simulink model "chapter2_5"  (R2022a) (continued)
== system chapter2_5/Subsystem3/Subsystem/Subsystem3/Subsystem13 ==
  index : Inport
  F : Inport  Port=2
  Add1 : Sum
  Add2 : Sum
  Constant2 : Constant
  Constant6 : Constant
  Constant7 : Constant
  Constant8 : Constant  Value=2
  Demux : Demux  Outputs=2
  Mux : Mux  Inputs=2
  Subsystem : Subsystem
  Subsystem1 : Subsystem
  Subsystem2 : Subsystem
  Subsystem3 : Subsystem
  Out1 : Outport
  wires:
  Mux/1 -> Out1/1
  Subsystem2/2(Out2) -> Add2/1
  Subsystem3/2(Out2) -> Add2/2
  Add2/1 -> Mux/2
  Subsystem3/1(Out1) -> Add1/2
  Subsystem2/1(Out1) -> Add1/1
  Subsystem1/8(Omiga_n) -> Subsystem3/8(Omiga_n)
  Subsystem1/7(Omiga_bn) -> Subsystem3/7(Omiga_bn)
  Subsystem1/6(static_n) -> Subsystem3/6(static_n)
  Subsystem1/5(Sn) -> Subsystem3/5(Sn)
  Subsystem1/4(theta_n3) -> Subsystem3/4(theta_n3)
  Subsystem1/3(theta_n2) -> Subsystem3/3(theta_n2)
  Subsystem1/2(theta_n1) -> Subsystem3/2(theta_n1)
  Subsystem1/1(An1) -> Subsystem3/1(An1)
  Subsystem/8(Omiga_n) -> Subsystem2/8(Omiga_n)
  Subsystem/7(Omiga_bn) -> Subsystem2/7(Omiga_bn)
  Subsystem/6(static_n) -> Subsystem2/6(static_n)
  Subsystem/5(Sn) -> Subsystem2/5(Sn)
  Subsystem/1(An1) -> Subsystem2/1(An1)
  Subsystem/4(theta_n3) -> Subsystem2/4(theta_n3)
  Subsystem/3(theta_n2) -> Subsystem2/3(theta_n2)
  Subsystem/2(theta_n1) -> Subsystem2/2(theta_n1)
  Demux/2 -> Subsystem1/4(F)
  Demux/1 -> Subsystem/4(F)
  F/1 -> Demux/1
  Constant7/1 -> Subsystem1/2(r)
  Constant8/1 -> Subsystem1/3(k)
  Constant6/1 -> Subsystem/3(k)
  Constant2/1 -> Subsystem/2(r)
  Add1/1 -> Mux/1
  index/1 -> Subsystem/1(imod), Subsystem1/1(imod)

== system chapter2_5/Subsystem3/Subsystem/Subsystem3/Subsystem14 ==
  index : Inport
  F : Inport  Port=2
  Add1 : Sum
  Add2 : Sum
  Constant2 : Constant
  Constant6 : Constant
  Constant7 : Constant
  Constant8 : Constant  Value=2
  Demux : Demux  Outputs=2
  Mux : Mux  Inputs=2
  Subsystem : Subsystem
  Subsystem1 : Subsystem
  Subsystem2 : Subsystem
  Subsystem3 : Subsystem
  Out1 : Outport
  wires:
  index/1 -> Subsystem1/1(imod), Subsystem/1(imod)
  Add1/1 -> Mux/1
  Constant2/1 -> Subsystem/2(r)
  Constant6/1 -> Subsystem/3(k)
  Constant8/1 -> Subsystem1/3(k)
  Constant7/1 -> Subsystem1/2(r)
  F/1 -> Demux/1
  Demux/1 -> Subsystem/4(F)
  Demux/2 -> Subsystem1/4(F)
  Subsystem/2(theta_n1) -> Subsystem2/2(theta_n1)
  Subsystem/3(theta_n2) -> Subsystem2/3(theta_n2)
  Subsystem/4(theta_n3) -> Subsystem2/4(theta_n3)
  Subsystem/1(An1) -> Subsystem2/1(An1)
  Subsystem/5(Sn) -> Subsystem2/5(Sn)
  Subsystem/6(static_n) -> Subsystem2/6(static_n)
  Subsystem/7(Omiga_bn) -> Subsystem2/7(Omiga_bn)
  Subsystem/8(Omiga_n) -> Subsystem2/8(Omiga_n)
  Subsystem1/1(An1) -> Subsystem3/1(An1)
  Subsystem1/2(theta_n1) -> Subsystem3/2(theta_n1)
  Subsystem1/3(theta_n2) -> Subsystem3/3(theta_n2)
  Subsystem1/4(theta_n3) -> Subsystem3/4(theta_n3)
  Subsystem1/5(Sn) -> Subsystem3/5(Sn)
  Subsystem1/6(static_n) -> Subsystem3/6(static_n)
  Subsystem1/7(Omiga_bn) -> Subsystem3/7(Omiga_bn)
  Subsystem1/8(Omiga_n) -> Subsystem3/8(Omiga_n)
  Subsystem2/1(Out1) -> Add1/1
  Subsystem3/1(Out1) -> Add1/2
  Add2/1 -> Mux/2
  Subsystem3/2(Out2) -> Add2/2
  Subsystem2/2(Out2) -> Add2/1
  Mux/1 -> Out1/1

== system chapter2_5/Subsystem3/Subsystem/Subsystem3/Subsystem15 ==
  index : Inport
  F : Inport  Port=2
  Add1 : Sum
  Add2 : Sum
  Constant2 : Constant
  Constant6 : Constant
  Constant7 : Constant
  Constant8 : Constant  Value=2
  Demux : Demux  Outputs=2
  Mux : Mux  Inputs=2
  Subsystem : Subsystem
  Subsystem1 : Subsystem
  Subsystem2 : Subsystem
  Subsystem3 : Subsystem
  Out1 : Outport
  wires:
  index/1 -> Subsystem1/1(imod), Subsystem/1(imod)
  Add1/1 -> Mux/1
  Constant2/1 -> Subsystem/2(r)
  Constant6/1 -> Subsystem/3(k)
  Constant8/1 -> Subsystem1/3(k)
  Constant7/1 -> Subsystem1/2(r)
  F/1 -> Demux/1
  Demux/1 -> Subsystem/4(F)
  Demux/2 -> Subsystem1/4(F)
  Subsystem/2(theta_n1) -> Subsystem2/2(theta_n1)
  Subsystem/3(theta_n2) -> Subsystem2/3(theta_n2)
  Subsystem/4(theta_n3) -> Subsystem2/4(theta_n3)
  Subsystem/1(An1) -> Subsystem2/1(An1)
  Subsystem/5(Sn) -> Subsystem2/5(Sn)
  Subsystem/6(static_n) -> Subsystem2/6(static_n)
  Subsystem/7(Omiga_bn) -> Subsystem2/7(Omiga_bn)
  Subsystem/8(Omiga_n) -> Subsystem2/8(Omiga_n)
  Subsystem1/1(An1) -> Subsystem3/1(An1)
  Subsystem1/2(theta_n1) -> Subsystem3/2(theta_n1)
  Subsystem1/3(theta_n2) -> Subsystem3/3(theta_n2)
  Subsystem1/4(theta_n3) -> Subsystem3/4(theta_n3)
  Subsystem1/5(Sn) -> Subsystem3/5(Sn)
  Subsystem1/6(static_n) -> Subsystem3/6(static_n)
  Subsystem1/7(Omiga_bn) -> Subsystem3/7(Omiga_bn)
  Subsystem1/8(Omiga_n) -> Subsystem3/8(Omiga_n)
  Subsystem2/1(Out1) -> Add1/1
  Subsystem3/1(Out1) -> Add1/2
  Add2/1 -> Mux/2
  Subsystem3/2(Out2) -> Add2/2
  Subsystem2/2(Out2) -> Add2/1
  Mux/1 -> Out1/1

== system chapter2_5/Subsystem3/Subsystem/Subsystem3/Subsystem16 ==
  index : Inport
  F : Inport  Port=2
  Add1 : Sum
  Add2 : Sum
  Constant2 : Constant
  Constant6 : Constant
  Constant7 : Constant
  Constant8 : Constant  Value=2
  Demux : Demux  Outputs=2
  Mux : Mux  Inputs=2
  Subsystem : Subsystem
  Subsystem1 : Subsystem
  Subsystem2 : Subsystem
  Subsystem3 : Subsystem
  Out1 : Outport
  wires:
  Mux/1 -> Out1/1
  Subsystem2/2(Out2) -> Add2/1
  Subsystem3/2(Out2) -> Add2/2
  Add2/1 -> Mux/2
  Subsystem3/1(Out1) -> Add1/2
  Subsystem2/1(Out1) -> Add1/1
  Subsystem1/8(Omiga_n) -> Subsystem3/8(Omiga_n)
  Subsystem1/7(Omiga_bn) -> Subsystem3/7(Omiga_bn)
  Subsystem1/6(static_n) -> Subsystem3/6(static_n)
  Subsystem1/5(Sn) -> Subsystem3/5(Sn)
  Subsystem1/4(theta_n3) -> Subsystem3/4(theta_n3)
  Subsystem1/3(theta_n2) -> Subsystem3/3(theta_n2)
  Subsystem1/2(theta_n1) -> Subsystem3/2(theta_n1)
  Subsystem1/1(An1) -> Subsystem3/1(An1)
  Subsystem/8(Omiga_n) -> Subsystem2/8(Omiga_n)
  Subsystem/7(Omiga_bn) -> Subsystem2/7(Omiga_bn)
  Subsystem/6(static_n) -> Subsystem2/6(static_n)
  Subsystem/5(Sn) -> Subsystem2/5(Sn)
  Subsystem/1(An1) -> Subsystem2/1(An1)
  Subsystem/4(theta_n3) -> Subsystem2/4(theta_n3)
  Subsystem/3(theta_n2) -> Subsystem2/3(theta_n2)
  Subsystem/2(theta_n1) -> Subsystem2/2(theta_n1)
  Demux/2 -> Subsystem1/4(F)
  Demux/1 -> Subsystem/4(F)
  F/1 -> Demux/1
  Constant7/1 -> Subsystem1/2(r)
  Constant8/1 -> Subsystem1/3(k)
  Constant6/1 -> Subsystem/3(k)
  Constant2/1 -> Subsystem/2(r)
  Add1/1 -> Mux/1
  index/1 -> Subsystem/1(imod), Subsystem1/1(imod)

== system chapter2_5/Subsystem3/Subsystem/Subsystem3/Subsystem17 ==
  index : Inport
  F : Inport  Port=2
  Add1 : Sum
  Add2 : Sum
  Constant2 : Constant
  Constant6 : Constant
  Constant7 : Constant
  Constant8 : Constant  Value=2
  Demux : Demux  Outputs=2
  Mux : Mux  Inputs=2
  Subsystem : Subsystem
  Subsystem1 : Subsystem
  Subsystem2 : Subsystem
  Subsystem3 : Subsystem
  Out1 : Outport
  wires:
  Mux/1 -> Out1/1
  Subsystem2/2(Out2) -> Add2/1
  Subsystem3/2(Out2) -> Add2/2
  Add2/1 -> Mux/2
  Subsystem3/1(Out1) -> Add1/2
  Subsystem2/1(Out1) -> Add1/1
  Subsystem1/8(Omiga_n) -> Subsystem3/8(Omiga_n)
  Subsystem1/7(Omiga_bn) -> Subsystem3/7(Omiga_bn)
  Subsystem1/6(static_n) -> Subsystem3/6(static_n)
  Subsystem1/5(Sn) -> Subsystem3/5(Sn)
  Subsystem1/4(theta_n3) -> Subsystem3/4(theta_n3)
  Subsystem1/3(theta_n2) -> Subsystem3/3(theta_n2)
  Subsystem1/2(theta_n1) -> Subsystem3/2(theta_n1)
  Subsystem1/1(An1) -> Subsystem3/1(An1)
  Subsystem/8(Omiga_n) -> Subsystem2/8(Omiga_n)
  Subsystem/7(Omiga_bn) -> Subsystem2/7(Omiga_bn)
  Subsystem/6(static_n) -> Subsystem2/6(static_n)
  Subsystem/5(Sn) -> Subsystem2/5(Sn)
  Subsystem/1(An1) -> Subsystem2/1(An1)
  Subsystem/4(theta_n3) -> Subsystem2/4(theta_n3)
  Subsystem/3(theta_n2) -> Subsystem2/3(theta_n2)
  Subsystem/2(theta_n1) -> Subsystem2/2(theta_n1)
  Demux/2 -> Subsystem1/4(F)
  Demux/1 -> Subsystem/4(F)
  F/1 -> Demux/1
  Constant7/1 -> Subsystem1/2(r)
  Constant8/1 -> Subsystem1/3(k)
  Constant6/1 -> Subsystem/3(k)
  Constant2/1 -> Subsystem/2(r)
  Add1/1 -> Mux/1
  index/1 -> Subsystem/1(imod), Subsystem1/1(imod)

== system chapter2_5/Subsystem3/Subsystem/Subsystem3/Subsystem18 ==
  index : Inport
  F : Inport  Port=2
  Add1 : Sum
  Add2 : Sum
  Constant2 : Constant
  Constant6 : Constant
  Constant7 : Constant
  Constant8 : Constant  Value=2
  Demux : Demux  Outputs=2
  Mux : Mux  Inputs=2
  Subsystem : Subsystem
  Subsystem1 : Subsystem
  Subsystem2 : Subsystem
  Subsystem3 : Subsystem
  Out1 : Outport
  wires:
  index/1 -> Subsystem1/1(imod), Subsystem/1(imod)
  Add1/1 -> Mux/1
  Constant2/1 -> Subsystem/2(r)
  Constant6/1 -> Subsystem/3(k)
  Constant8/1 -> Subsystem1/3(k)
  Constant7/1 -> Subsystem1/2(r)
  F/1 -> Demux/1
  Demux/1 -> Subsystem/4(F)
  Demux/2 -> Subsystem1/4(F)
  Subsystem/2(theta_n1) -> Subsystem2/2(theta_n1)
  Subsystem/3(theta_n2) -> Subsystem2/3(theta_n2)
  Subsystem/4(theta_n3) -> Subsystem2/4(theta_n3)
  Subsystem/1(An1) -> Subsystem2/1(An1)
  Subsystem/5(Sn) -> Subsystem2/5(Sn)
  Subsystem/6(static_n) -> Subsystem2/6(static_n)
  Subsystem/7(Omiga_bn) -> Subsystem2/7(Omiga_bn)
  Subsystem/8(Omiga_n) -> Subsystem2/8(Omiga_n)
  Subsystem1/1(An1) -> Subsystem3/1(An1)
  Subsystem1/2(theta_n1) -> Subsystem3/2(theta_n1)
  Subsystem1/3(theta_n2) -> Subsystem3/3(theta_n2)
  Subsystem1/4(theta_n3) -> Subsystem3/4(theta_n3)
  Subsystem1/5(Sn) -> Subsystem3/5(Sn)
  Subsystem1/6(static_n) -> Subsystem3/6(static_n)
  Subsystem1/7(Omiga_bn) -> Subsystem3/7(Omiga_bn)
  Subsystem1/8(Omiga_n) -> Subsystem3/8(Omiga_n)
  Subsystem2/1(Out1) -> Add1/1
  Subsystem3/1(Out1) -> Add1/2
  Add2/1 -> Mux/2
  Subsystem3/2(Out2) -> Add2/2
  Subsystem2/2(Out2) -> Add2/1
  Mux/1 -> Out1/1

== system chapter2_5/Subsystem3/Subsystem/Subsystem3/Subsystem19 ==
  index : Inport
  F : Inport  Port=2
  Add1 : Sum
  Add2 : Sum
  Constant2 : Constant
  Constant6 : Constant
  Constant7 : Constant
  Constant8 : Constant  Value=2
  Demux : Demux  Outputs=2
  Mux : Mux  Inputs=2
  Subsystem : Subsystem
  Subsystem1 : Subsystem
  Subsystem2 : Subsystem
  Subsystem3 : Subsystem
  Out1 : Outport
  wires:
  Mux/1 -> Out1/1
  Subsystem2/2(Out2) -> Add2/1
  Subsystem3/2(Out2) -> Add2/2
  Add2/1 -> Mux/2
  Subsystem3/1(Out1) -> Add1/2
  Subsystem2/1(Out1) -> Add1/1
  Subsystem1/8(Omiga_n) -> Subsystem3/8(Omiga_n)
  Subsystem1/7(Omiga_bn) -> Subsystem3/7(Omiga_bn)
  Subsystem1/6(static_n) -> Subsystem3/6(static_n)
  Subsystem1/5(Sn) -> Subsystem3/5(Sn)
  Subsystem1/4(theta_n3) -> Subsystem3/4(theta_n3)
  Subsystem1/3(theta_n2) -> Subsystem3/3(theta_n2)
  Subsystem1/2(theta_n1) -> Subsystem3/2(theta_n1)
  Subsystem1/1(An1) -> Subsystem3/1(An1)
  Subsystem/8(Omiga_n) -> Subsystem2/8(Omiga_n)
  Subsystem/7(Omiga_bn) -> Subsystem2/7(Omiga_bn)
  Subsystem/6(static_n) -> Subsystem2/6(static_n)
  Subsystem/5(Sn) -> Subsystem2/5(Sn)
  Subsystem/1(An1) -> Subsystem2/1(An1)
  Subsystem/4(theta_n3) -> Subsystem2/4(theta_n3)
  Subsystem/3(theta_n2) -> Subsystem2/3(theta_n2)
  Subsystem/2(theta_n1) -> Subsystem2/2(theta_n1)
  Demux/2 -> Subsystem1/4(F)
  Demux/1 -> Subsystem/4(F)
  F/1 -> Demux/1
  Constant7/1 -> Subsystem1/2(r)
  Constant8/1 -> Subsystem1/3(k)
  Constant6/1 -> Subsystem/3(k)
  Constant2/1 -> Subsystem/2(r)
  Add1/1 -> Mux/1
  index/1 -> Subsystem/1(imod), Subsystem1/1(imod)

== system chapter2_5/Subsystem3/Subsystem/Subsystem3/Subsystem2 ==
  index : Inport
  F : Inport  Port=2
  Add1 : Sum
  Add2 : Sum
  Constant2 : Constant
  Constant6 : Constant
  Constant7 : Constant
  Constant8 : Constant  Value=2
  Demux : Demux  Outputs=2
  Mux : Mux  Inputs=2
  Subsystem : Subsystem
  Subsystem1 : Subsystem
  Subsystem2 : Subsystem
  Subsystem3 : Subsystem
  Out1 : Outport
  wires:
  Mux/1 -> Out1/1
  Subsystem2/2(Out2) -> Add2/1
  Subsystem3/2(Out2) -> Add2/2
  Add2/1 -> Mux/2
  Subsystem3/1(Out1) -> Add1/2
  Subsystem2/1(Out1) -> Add1/1
  Subsystem1/8(Omiga_n) -> Subsystem3/8(Omiga_n)
  Subsystem1/7(Omiga_bn) -> Subsystem3/7(Omiga_bn)
  Subsystem1/6(static_n) -> Subsystem3/6(static_n)
  Subsystem1/5(Sn) -> Subsystem3/5(Sn)
  Subsystem1/4(theta_n3) -> Subsystem3/4(theta_n3)
  Subsystem1/3(theta_n2) -> Subsystem3/3(theta_n2)
  Subsystem1/2(theta_n1) -> Subsystem3/2(theta_n1)
  Subsystem1/1(An1) -> Subsystem3/1(An1)
  Subsystem/8(Omiga_n) -> Subsystem2/8(Omiga_n)
  Subsystem/7(Omiga_bn) -> Subsystem2/7(Omiga_bn)
  Subsystem/6(static_n) -> Subsystem2/6(static_n)
  Subsystem/5(Sn) -> Subsystem2/5(Sn)
  Subsystem/1(An1) -> Subsystem2/1(An1)
  Subsystem/4(theta_n3) -> Subsystem2/4(theta_n3)
  Subsystem/3(theta_n2) -> Subsystem2/3(theta_n2)
  Subsystem/2(theta_n1) -> Subsystem2/2(theta_n1)
  Demux/2 -> Subsystem1/4(F)
  Demux/1 -> Subsystem/4(F)
  F/1 -> Demux/1
  Constant7/1 -> Subsystem1/2(r)
  Constant8/1 -> Subsystem1/3(k)
  Constant6/1 -> Subsystem/3(k)
  Constant2/1 -> Subsystem/2(r)
  Add1/1 -> Mux/1
  index/1 -> Subsystem/1(imod), Subsystem1/1(imod)

== system chapter2_5/Subsystem3/Subsystem/Subsystem3/Subsystem20 ==
  index : Inport
  F : Inport  Port=2
  Add1 : Sum
  Add2 : Sum
  Constant2 : Constant
  Constant6 : Constant
  Constant7 : Constant
  Constant8 : Constant  Value=2
  Demux : Demux  Outputs=2
  Mux : Mux  Inputs=2
  Subsystem : Subsystem
  Subsystem1 : Subsystem
  Subsystem2 : Subsystem
  Subsystem3 : Subsystem
  Out1 : Outport
  wires:
  index/1 -> Subsystem1/1(imod), Subsystem/1(imod)
  Add1/1 -> Mux/1
  Constant2/1 -> Subsystem/2(r)
  Constant6/1 -> Subsystem/3(k)
  Constant8/1 -> Subsystem1/3(k)
  Constant7/1 -> Subsystem1/2(r)
  F/1 -> Demux/1
  Demux/1 -> Subsystem/4(F)
  Demux/2 -> Subsystem1/4(F)
  Subsystem/2(theta_n1) -> Subsystem2/2(theta_n1)
  Subsystem/3(theta_n2) -> Subsystem2/3(theta_n2)
  Subsystem/4(theta_n3) -> Subsystem2/4(theta_n3)
  Subsystem/1(An1) -> Subsystem2/1(An1)
  Subsystem/5(Sn) -> Subsystem2/5(Sn)
  Subsystem/6(static_n) -> Subsystem2/6(static_n)
  Subsystem/7(Omiga_bn) -> Subsystem2/7(Omiga_bn)
  Subsystem/8(Omiga_n) -> Subsystem2/8(Omiga_n)
  Subsystem1/1(An1) -> Subsystem3/1(An1)
  Subsystem1/2(theta_n1) -> Subsystem3/2(theta_n1)
  Subsystem1/3(theta_n2) -> Subsystem3/3(theta_n2)
  Subsystem1/4(theta_n3) -> Subsystem3/4(theta_n3)
  Subsystem1/5(Sn) -> Subsystem3/5(Sn)
  Subsystem1/6(static_n) -> Subsystem3/6(static_n)
  Subsystem1/7(Omiga_bn) -> Subsystem3/7(Omiga_bn)
  Subsystem1/8(Omiga_n) -> Subsystem3/8(Omiga_n)
  Subsystem2/1(Out1) -> Add1/1
  Subsystem3/1(Out1) -> Add1/2
  Add2/1 -> Mux/2
  Subsystem3/2(Out2) -> Add2/2
  Subsystem2/2(Out2) -> Add2/1
  Mux/1 -> Out1/1

== system chapter2_5/Subsystem3/Subsystem/Subsystem3/Subsystem3 ==
  index : Inport
  F : Inport  Port=2
  Add1 : Sum
  Add2 : Sum
  Constant2 : Constant
  Constant6 : Constant
  Constant7 : Constant
  Constant8 : Constant  Value=2
  Demux : Demux  Outputs=2
  Mux : Mux  Inputs=2
  Subsystem : Subsystem
  Subsystem1 : Subsystem
  Subsystem2 : Subsystem
  Subsystem3 : Subsystem
  Out1 : Outport
  wires:
  index/1 -> Subsystem1/1(imod), Subsystem/1(imod)
  Add1/1 -> Mux/1
  Constant2/1 -> Subsystem/2(r)
  Constant6/1 -> Subsystem/3(k)
  Constant8/1 -> Subsystem1/3(k)
  Constant7/1 -> Subsystem1/2(r)
  F/1 -> Demux/1
  Demux/1 -> Subsystem/4(F)
  Demux/2 -> Subsystem1/4(F)
  Subsystem/2(theta_n1) -> Subsystem2/2(theta_n1)
  Subsystem/3(theta_n2) -> Subsystem2/3(theta_n2)
  Subsystem/4(theta_n3) -> Subsystem2/4(theta_n3)
  Subsystem/1(An1) -> Subsystem2/1(An1)
  Subsystem/5(Sn) -> Subsystem2/5(Sn)
  Subsystem/6(static_n) -> Subsystem2/6(static_n)
  Subsystem/7(Omiga_bn) -> Subsystem2/7(Omiga_bn)
  Subsystem/8(Omiga_n) -> Subsystem2/8(Omiga_n)
  Subsystem1/1(An1) -> Subsystem3/1(An1)
  Subsystem1/2(theta_n1) -> Subsystem3/2(theta_n1)
  Subsystem1/3(theta_n2) -> Subsystem3/3(theta_n2)
  Subsystem1/4(theta_n3) -> Subsystem3/4(theta_n3)
  Subsystem1/5(Sn) -> Subsystem3/5(Sn)
  Subsystem1/6(static_n) -> Subsystem3/6(static_n)
  Subsystem1/7(Omiga_bn) -> Subsystem3/7(Omiga_bn)
  Subsystem1/8(Omiga_n) -> Subsystem3/8(Omiga_n)
  Subsystem2/1(Out1) -> Add1/1
  Subsystem3/1(Out1) -> Add1/2
  Add2/1 -> Mux/2
  Subsystem3/2(Out2) -> Add2/2
  Subsystem2/2(Out2) -> Add2/1
  Mux/1 -> Out1/1

== system chapter2_5/Subsystem3/Subsystem/Subsystem3/Subsystem4 ==
  index : Inport
  F : Inport  Port=2
  Add1 : Sum
  Add2 : Sum
  Constant2 : Constant
  Constant6 : Constant
  Constant7 : Constant
  Constant8 : Constant  Value=2
  Demux : Demux  Outputs=2
  Mux : Mux  Inputs=2
  Subsystem : Subsystem
  Subsystem1 : Subsystem
  Subsystem2 : Subsystem
  Subsystem3 : Subsystem
  Out1 : Outport
  wires:
  Mux/1 -> Out1/1
  Subsystem2/2(Out2) -> Add2/1
  Subsystem3/2(Out2) -> Add2/2
  Add2/1 -> Mux/2
  Subsystem3/1(Out1) -> Add1/2
  Subsystem2/1(Out1) -> Add1/1
  Subsystem1/8(Omiga_n) -> Subsystem3/8(Omiga_n)
  Subsystem1/7(Omiga_bn) -> Subsystem3/7(Omiga_bn)
  Subsystem1/6(static_n) -> Subsystem3/6(static_n)
  Subsystem1/5(Sn) -> Subsystem3/5(Sn)
  Subsystem1/4(theta_n3) -> Subsystem3/4(theta_n3)
  Subsystem1/3(theta_n2) -> Subsystem3/3(theta_n2)
  Subsystem1/2(theta_n1) -> Subsystem3/2(theta_n1)
  Subsystem1/1(An1) -> Subsystem3/1(An1)
  Subsystem/8(Omiga_n) -> Subsystem2/8(Omiga_n)
  Subsystem/7(Omiga_bn) -> Subsystem2/7(Omiga_bn)
  Subsystem/6(static_n) -> Subsystem2/6(static_n)
  Subsystem/5(Sn) -> Subsystem2/5(Sn)
  Subsystem/1(An1) -> Subsystem2/1(An1)
  Subsystem/4(theta_n3) -> Subsystem2/4(theta_n3)
  Subsystem/3(theta_n2) -> Subsystem2/3(theta_n2)
  Subsystem/2(theta_n1) -> Subsystem2/2(theta_n1)
  Demux/2 -> Subsystem1/4(F)
  Demux/1 -> Subsystem/4(F)
  F/1 -> Demux/1
  Constant7/1 -> Subsystem1/2(r)
  Constant8/1 -> Subsystem1/3(k)
  Constant6/1 -> Subsystem/3(k)
  Constant2/1 -> Subsystem/2(r)
  Add1/1 -> Mux/1
  index/1 -> Subsystem/1(imod), Subsystem1/1(imod)

== system chapter2_5/Subsystem3/Subsystem/Subsystem3/Subsystem5 ==
  index : Inport
  F : Inport  Port=2
  Add1 : Sum
  Add2 : Sum
  Constant2 : Constant
  Constant6 : Constant
  Constant7 : Constant
  Constant8 : Constant  Value=2
  Demux : Demux  Outputs=2
  Mux : Mux  Inputs=2
  Subsystem : Subsystem
  Subsystem1 : Subsystem
  Subsystem2 : Subsystem
  Subsystem3 : Subsystem
  Out1 : Outport
  wires:
  index/1 -> Subsystem1/1(imod), Subsystem/1(imod)
  Add1/1 -> Mux/1
  Constant2/1 -> Subsystem/2(r)
  Constant6/1 -> Subsystem/3(k)
  Constant8/1 -> Subsystem1/3(k)
  Constant7/1 -> Subsystem1/2(r)
  F/1 -> Demux/1
  Demux/1 -> Subsystem/4(F)
  Demux/2 -> Subsystem1/4(F)
  Subsystem/2(theta_n1) -> Subsystem2/2(theta_n1)
  Subsystem/3(theta_n2) -> Subsystem2/3(theta_n2)
  Subsystem/4(theta_n3) -> Subsystem2/4(theta_n3)
  Subsystem/1(An1) -> Subsystem2/1(An1)
  Subsystem/5(Sn) -> Subsystem2/5(Sn)
  Subsystem/6(static_n) -> Subsystem2/6(static_n)
  Subsystem/7(Omiga_bn) -> Subsystem2/7(Omiga_bn)
  Subsystem/8(Omiga_n) -> Subsystem2/8(Omiga_n)
  Subsystem1/1(An1) -> Subsystem3/1(An1)
  Subsystem1/2(theta_n1) -> Subsystem3/2(theta_n1)
  Subsystem1/3(theta_n2) -> Subsystem3/3(theta_n2)
  Subsystem1/4(theta_n3) -> Subsystem3/4(theta_n3)
  Subsystem1/5(Sn) -> Subsystem3/5(Sn)
  Subsystem1/6(static_n) -> Subsystem3/6(static_n)
  Subsystem1/7(Omiga_bn) -> Subsystem3/7(Omiga_bn)
  Subsystem1/8(Omiga_n) -> Subsystem3/8(Omiga_n)
  Subsystem2/1(Out1) -> Add1/1
  Subsystem3/1(Out1) -> Add1/2
  Add2/1 -> Mux/2
  Subsystem3/2(Out2) -> Add2/2
  Subsystem2/2(Out2) -> Add2/1
  Mux/1 -> Out1/1

== system chapter2_5/Subsystem3/Subsystem/Subsystem3/Subsystem6 ==
  index : Inport
  F : Inport  Port=2
  Add1 : Sum
  Add2 : Sum
  Constant2 : Constant
  Constant6 : Constant
  Constant7 : Constant
  Constant8 : Constant  Value=2
  Demux : Demux  Outputs=2
  Mux : Mux  Inputs=2
  Subsystem : Subsystem
  Subsystem1 : Subsystem
  Subsystem2 : Subsystem
  Subsystem3 : Subsystem
  Out1 : Outport
  wires:
  Mux/1 -> Out1/1
  Subsystem2/2(Out2) -> Add2/1
  Subsystem3/2(Out2) -> Add2/2
  Add2/1 -> Mux/2
  Subsystem3/1(Out1) -> Add1/2
  Subsystem2/1(Out1) -> Add1/1
  Subsystem1/8(Omiga_n) -> Subsystem3/8(Omiga_n)
  Subsystem1/7(Omiga_bn) -> Subsystem3/7(Omiga_bn)
  Subsystem1/6(static_n) -> Subsystem3/6(static_n)
  Subsystem1/5(Sn) -> Subsystem3/5(Sn)
  Subsystem1/4(theta_n3) -> Subsystem3/4(theta_n3)
  Subsystem1/3(theta_n2) -> Subsystem3/3(theta_n2)
  Subsystem1/2(theta_n1) -> Subsystem3/2(theta_n1)
  Subsystem1/1(An1) -> Subsystem3/1(An1)
  Subsystem/8(Omiga_n) -> Subsystem2/8(Omiga_n)
  Subsystem/7(Omiga_bn) -> Subsystem2/7(Omiga_bn)
  Subsystem/6(static_n) -> Subsystem2/6(static_n)
  Subsystem/5(Sn) -> Subsystem2/5(Sn)
  Subsystem/1(An1) -> Subsystem2/1(An1)
  Subsystem/4(theta_n3) -> Subsystem2/4(theta_n3)
  Subsystem/3(theta_n2) -> Subsystem2/3(theta_n2)
  Subsystem/2(theta_n1) -> Subsystem2/2(theta_n1)
  Demux/2 -> Subsystem1/4(F)
  Demux/1 -> Subsystem/4(F)
  F/1 -> Demux/1
  Constant7/1 -> Subsystem1/2(r)
  Constant8/1 -> Subsystem1/3(k)
  Constant6/1 -> Subsystem/3(k)
  Constant2/1 -> Subsystem/2(r)
  Add1/1 -> Mux/1
  index/1 -> Subsystem/1(imod), Subsystem1/1(imod)

== system chapter2_5/Subsystem3/Subsystem/Subsystem3/Subsystem7 ==
  index : Inport
  F : Inport  Port=2
  Add1 : Sum
  Add2 : Sum
  Constant2 : Constant
  Constant6 : Constant
  Constant7 : Constant
  Constant8 : Constant  Value=2
  Demux : Demux  Outputs=2
  Mux : Mux  Inputs=2
  Subsystem : Subsystem
  Subsystem1 : Subsystem
  Subsystem2 : Subsystem
  Subsystem3 : Subsystem
  Out1 : Outport
  wires:
  Mux/1 -> Out1/1
  Subsystem2/2(Out2) -> Add2/1
  Subsystem3/2(Out2) -> Add2/2
  Add2/1 -> Mux/2
  Subsystem3/1(Out1) -> Add1/2
  Subsystem2/1(Out1) -> Add1/1
  Subsystem1/8(Omiga_n) -> Subsystem3/8(Omiga_n)
  Subsystem1/7(Omiga_bn) -> Subsystem3/7(Omiga_bn)
  Subsystem1/6(static_n) -> Subsystem3/6(static_n)
  Subsystem1/5(Sn) -> Subsystem3/5(Sn)
  Subsystem1/4(theta_n3) -> Subsystem3/4(theta_n3)
  Subsystem1/3(theta_n2) -> Subsystem3/3(theta_n2)
  Subsystem1/2(theta_n1) -> Subsystem3/2(theta_n1)
  Subsystem1/1(An1) -> Subsystem3/1(An1)
  Subsystem/8(Omiga_n) -> Subsystem2/8(Omiga_n)
  Subsystem/7(Omiga_bn) -> Subsystem2/7(Omiga_bn)
  Subsystem/6(static_n) -> Subsystem2/6(static_n)
  Subsystem/5(Sn) -> Subsystem2/5(Sn)
  Subsystem/1(An1) -> Subsystem2/1(An1)
  Subsystem/4(theta_n3) -> Subsystem2/4(theta_n3)
  Subsystem/3(theta_n2) -> Subsystem2/3(theta_n2)
  Subsystem/2(theta_n1) -> Subsystem2/2(theta_n1)
  Demux/2 -> Subsystem1/4(F)
  Demux/1 -> Subsystem/4(F)
  F/1 -> Demux/1
  Constant7/1 -> Subsystem1/2(r)
  Constant8/1 -> Subsystem1/3(k)
  Constant6/1 -> Subsystem/3(k)
  Constant2/1 -> Subsystem/2(r)
  Add1/1 -> Mux/1
  index/1 -> Subsystem/1(imod), Subsystem1/1(imod)

== system chapter2_5/Subsystem3/Subsystem/Subsystem3/Subsystem8 ==
  index : Inport
  F : Inport  Port=2
  Add1 : Sum
  Add2 : Sum
  Constant2 : Constant
  Constant6 : Constant
  Constant7 : Constant
  Constant8 : Constant  Value=2
  Demux : Demux  Outputs=2
  Mux : Mux  Inputs=2
  Subsystem : Subsystem
  Subsystem1 : Subsystem
  Subsystem2 : Subsystem
  Subsystem3 : Subsystem
  Out1 : Outport
  wires:
  index/1 -> Subsystem1/1(imod), Subsystem/1(imod)
  Add1/1 -> Mux/1
  Constant2/1 -> Subsystem/2(r)
  Constant6/1 -> Subsystem/3(k)
  Constant8/1 -> Subsystem1/3(k)
  Constant7/1 -> Subsystem1/2(r)
  F/1 -> Demux/1
  Demux/1 -> Subsystem/4(F)
  Demux/2 -> Subsystem1/4(F)
  Subsystem/2(theta_n1) -> Subsystem2/2(theta_n1)
  Subsystem/3(theta_n2) -> Subsystem2/3(theta_n2)
  Subsystem/4(theta_n3) -> Subsystem2/4(theta_n3)
  Subsystem/1(An1) -> Subsystem2/1(An1)
  Subsystem/5(Sn) -> Subsystem2/5(Sn)
  Subsystem/6(static_n) -> Subsystem2/6(static_n)
  Subsystem/7(Omiga_bn) -> Subsystem2/7(Omiga_bn)
  Subsystem/8(Omiga_n) -> Subsystem2/8(Omiga_n)
  Subsystem1/1(An1) -> Subsystem3/1(An1)
  Subsystem1/2(theta_n1) -> Subsystem3/2(theta_n1)
  Subsystem1/3(theta_n2) -> Subsystem3/3(theta_n2)
  Subsystem1/4(theta_n3) -> Subsystem3/4(theta_n3)
  Subsystem1/5(Sn) -> Subsystem3/5(Sn)
  Subsystem1/6(static_n) -> Subsystem3/6(static_n)
  Subsystem1/7(Omiga_bn) -> Subsystem3/7(Omiga_bn)
  Subsystem1/8(Omiga_n) -> Subsystem3/8(Omiga_n)
  Subsystem2/1(Out1) -> Add1/1
  Subsystem3/1(Out1) -> Add1/2
  Add2/1 -> Mux/2
  Subsystem3/2(Out2) -> Add2/2
  Subsystem2/2(Out2) -> Add2/1
  Mux/1 -> Out1/1

== system chapter2_5/Subsystem3/Subsystem/Subsystem3/Subsystem9 ==
  index : Inport
  F : Inport  Port=2
  Add1 : Sum
  Add2 : Sum
  Constant2 : Constant
  Constant6 : Constant
  Constant7 : Constant
  Constant8 : Constant  Value=2
  Demux : Demux  Outputs=2
  Mux : Mux  Inputs=2
  Subsystem : Subsystem
  Subsystem1 : Subsystem
  Subsystem2 : Subsystem
  Subsystem3 : Subsystem
  Out1 : Outport
  wires:
  Mux/1 -> Out1/1
  Subsystem2/2(Out2) -> Add2/1
  Subsystem3/2(Out2) -> Add2/2
  Add2/1 -> Mux/2
  Subsystem3/1(Out1) -> Add1/2
  Subsystem2/1(Out1) -> Add1/1
  Subsystem1/8(Omiga_n) -> Subsystem3/8(Omiga_n)
  Subsystem1/7(Omiga_bn) -> Subsystem3/7(Omiga_bn)
  Subsystem1/6(static_n) -> Subsystem3/6(static_n)
  Subsystem1/5(Sn) -> Subsystem3/5(Sn)
  Subsystem1/4(theta_n3) -> Subsystem3/4(theta_n3)
  Subsystem1/3(theta_n2) -> Subsystem3/3(theta_n2)
  Subsystem1/2(theta_n1) -> Subsystem3/2(theta_n1)
  Subsystem1/1(An1) -> Subsystem3/1(An1)
  Subsystem/8(Omiga_n) -> Subsystem2/8(Omiga_n)
  Subsystem/7(Omiga_bn) -> Subsystem2/7(Omiga_bn)
  Subsystem/6(static_n) -> Subsystem2/6(static_n)
  Subsystem/5(Sn) -> Subsystem2/5(Sn)
  Subsystem/1(An1) -> Subsystem2/1(An1)
  Subsystem/4(theta_n3) -> Subsystem2/4(theta_n3)
  Subsystem/3(theta_n2) -> Subsystem2/3(theta_n2)
  Subsystem/2(theta_n1) -> Subsystem2/2(theta_n1)
  Demux/2 -> Subsystem1/4(F)
  Demux/1 -> Subsystem/4(F)
  F/1 -> Demux/1
  Constant7/1 -> Subsystem1/2(r)
  Constant8/1 -> Subsystem1/3(k)
  Constant6/1 -> Subsystem/3(k)
  Constant2/1 -> Subsystem/2(r)
  Add1/1 -> Mux/1
  index/1 -> Subsystem/1(imod), Subsystem1/1(imod)
